simulink model "VirtualADAPT"  (Simulink 8.9)
settings: Solver=ode45  StartTime=0.0  StopTime=inf  RelTol=1e-3  EnableMultiTasking=on
PostLoadFcn:
  | FaultInjectionGUIHandle = FaultInjectionGUI;
InitFcn:
  | VirtualADAPTDataStructure
undefined symbols (not defined in the file or in its folder): ln
libraries: simulink x363
(part 14 of 26)

== system VirtualADAPT/VirtualADAPTv1/PowerStorage/Battery2Subsystem/CircuitBreakerEY236/Delay ==
  Input : Inport
  C : Subsystem  mask{C=0.8, IC=0, FP=Nominal, FT=0, FM=0}
      mask prompts: C "Value of C:"; IC "Initial Condition:"; FP "Fault Profile:"; FT "Fault Injection Time:"; FM "Fault Magnitude/Slope"
      mask TypeOptions FP: Nominal | Abrupt | Incipient
      mask Display: image(imread('C.png','BackgroundColor',[1 1 1]))
  Function : Subsystem
      mask Display: image(imread('fx.png','BackgroundColor',[1 1 1]))
  OneJunction : Subsystem
      mask Display: image(imread('1.png','BackgroundColor',[1 1 1]))
  R : Subsystem  mask{R=1, FP=Nominal, FT=0, FM=0}
      mask prompts: R "Value of R:"; FP "Fault Profile:"; FT "Fault Injection Time:"; FM "Fault Magnitude/Slope"
      mask TypeOptions FP: Nominal | Abrupt | Incipient
      mask Display: image(imread('R.png','BackgroundColor',[1 1 1]))
  Se : Subsystem  mask{FP=Nominal, FT=0, FM=0}
      mask prompts: FP "Fault Profile:"; FT "Fault Injection Time:"; FM "Fault Magnitude/Slope/Bias/StuckAtValue"
      mask TypeOptions FP: Nominal | Abrupt | Incipient | Bias | StuckAt
      mask Display: image(imread('MSe.png','BackgroundColor',[1 1 1]))
  ZeroJunction : Subsystem
      mask Display: image(imread('0.png','BackgroundColor',[1 1 1]))
  Output : Outport
  wires:
  OneJunction/1(y(1)) -> R/1(In)
  R/1(Out) -> OneJunction/1(u(1))
  Se/1(E) -> OneJunction/2(u(2))
  OneJunction/2(y(2)) -> Se/1(F)
  ZeroJunction/1(y(1)) -> C/1(F)
  C/1(E) -> ZeroJunction/1(u(1))
  OneJunction/3(y(3)) -> ZeroJunction/2(u(2))
  ZeroJunction/2(y(2)) -> OneJunction/3(u(3))
  Function/1(y(1)) -> Se/2(Se)
  Input/1 -> Function/1(u(1))
  ZeroJunction/3(O) -> Output/1

== system VirtualADAPT/VirtualADAPTv1/PowerStorage/Battery2Subsystem/CircuitBreakerEY236/ParallelConn ==
  u(1) : Inport
  u(2) : Inport  Port=2
  u(3) : Inport  Port=3
  u(4) : Inport  Port=4
  u(5) : Inport  Port=5
  Adder : Sum  Inputs=--  SaturateOnIntegerOverflow=on  InputSameDT=on  OutDataTypeStr=Inherit: Same as first input
  Gain : Gain  Gain=-1  SaturateOnIntegerOverflow=on  OutDataTypeStr=Inherit: Same as input  ParamDataTypeStr=Inherit: Same as input
  Mux : Mux  Inputs=5
  OffGuard : Fcn  Expr=u(5)
  OnGuard : Fcn  Expr=u(4)
  Product1 : Product  SaturateOnIntegerOverflow=on  RndMeth=Zero  InputSameDT=on  OutDataTypeStr=Inherit: Same as first input
  Product2 : Product  SaturateOnIntegerOverflow=on  RndMeth=Zero  InputSameDT=on  OutDataTypeStr=Inherit: Same as first input
  determineState : Subsystem
  y(1) : Outport
  y(2) : Outport  Port=2
  y(3) : Outport  Port=3
  O : Outport  Port=4
  State : Outport  Port=5
  wires:
  Adder/1 -> Gain/1
  u(4)/1 -> Mux/4
  u(5)/1 -> Mux/5
  Mux/1 -> OnGuard/1, OffGuard/1
  OnGuard/1 -> determineState/1(In1)
  OffGuard/1 -> determineState/2(In2)
  u(1)/1 -> Product1/1
  determineState/1(Out1) -> Product1/2, Product2/2, State/1
  Product1/1 -> O/1, y(2)/1, y(3)/1
  Gain/1 -> Product2/1
  Product2/1 -> y(1)/1
  u(2)/1 -> Adder/1
  u(3)/1 -> Adder/2

== system VirtualADAPT/VirtualADAPTv1/PowerStorage/Battery2Subsystem/CircuitBreakerEY236/R ==
  In : Inport
  Bond : Constant  Value=2
  Computing R : Subsystem
  Fault Profile : Constant  Value=FP
  JuncDB : Constant  Value=3
  Junction Type : Constant  Value=0
  Ramp : simulink/Sources/Ramp  slope=FM  start=FT  X0=R
  Resistor Value : Constant  Value=R
  Step : Step  Time=FT  Before=R  After=(1 + FM)*R  SampleTime=0
  Out : Outport
  R : Outport  Port=2
  wires:
  Computing R/1(Out1) -> Out/1
  In/1 -> Computing R/1(In1)
  Resistor Value/1 -> Computing R/2(RNominal)
  Fault Profile/1 -> Computing R/3(Fault Profile)
  Step/1 -> Computing R/4(Step)
  Ramp/1 -> Computing R/5(Ramp)
  JuncDB/1 -> Computing R/6(JuncDB)
  Bond/1 -> Computing R/7(Bond)
  Junction Type/1 -> Computing R/8(Junction Type)
  Computing R/2(Out2) -> R/1

== system VirtualADAPT/VirtualADAPTv1/PowerStorage/Battery2Subsystem/CircuitBreakerEY236/ZeroJunc ==
  u(1) : Inport
  u(2) : Inport  Port=2
  u(3) : Inport  Port=3
  Adder : Sum  Inputs=+-  SaturateOnIntegerOverflow=on  InputSameDT=on  OutDataTypeStr=Inherit: Same as first input
  Gain : Gain  SaturateOnIntegerOverflow=on  OutDataTypeStr=Inherit: Same as input  ParamDataTypeStr=Inherit: Same as input
  y(1) : Outport
  y(2) : Outport  Port=2
  y(3) : Outport  Port=3
  O : Outport  Port=4
  wires:
  Adder/1 -> Gain/1
  u(3)/1 -> y(1)/1, y(2)/1, O/1
  u(1)/1 -> Adder/1
  u(2)/1 -> Adder/2
  Gain/1 -> y(3)/1

== system VirtualADAPT/VirtualADAPTv1/PowerStorage/Battery2Subsystem/RelayEY241/ParallelConn ==
  u(1) : Inport
  u(2) : Inport  Port=2
  u(3) : Inport  Port=3
  Adder : Sum  Inputs=-  SaturateOnIntegerOverflow=on  InputSameDT=on  OutDataTypeStr=Inherit: Same as first input
  Gain : Gain  Gain=-1  SaturateOnIntegerOverflow=on  OutDataTypeStr=Inherit: Same as input  ParamDataTypeStr=Inherit: Same as input
  Mux : Mux  Inputs=3
  OffGuard : Fcn  Expr=(!u(3))
  OnGuard : Fcn  Expr=(u(3))
  Product1 : Product  SaturateOnIntegerOverflow=on  RndMeth=Zero  InputSameDT=on  OutDataTypeStr=Inherit: Same as first input
  Product2 : Product  SaturateOnIntegerOverflow=on  RndMeth=Zero  InputSameDT=on  OutDataTypeStr=Inherit: Same as first input
  determineState : Subsystem
  y(1) : Outport
  y(2) : Outport  Port=2
  O : Outport  Port=3
  State : Outport  Port=4
  wires:
  Adder/1 -> Gain/1
  u(3)/1 -> Mux/3
  Mux/1 -> OnGuard/1, OffGuard/1
  OnGuard/1 -> determineState/1(In1)
  OffGuard/1 -> determineState/2(In2)
  u(1)/1 -> Product1/1
  determineState/1(Out1) -> Product1/2, Product2/2, State/1
  Product1/1 -> O/1, y(2)/1
  Gain/1 -> Product2/1
  Product2/1 -> y(1)/1
  u(2)/1 -> Adder/1

== system VirtualADAPT/VirtualADAPTv1/PowerStorage/Battery2Subsystem/RelayEY241/Relay ==
  u(1) : Inport
  u(2) : Inport  Port=2
  u(3) : Inport  Port=3
  Adder : Sum  Inputs=+  SaturateOnIntegerOverflow=on  InputSameDT=on  OutDataTypeStr=Inherit: Same as first input
  Gain : Gain  SaturateOnIntegerOverflow=on  OutDataTypeStr=Inherit: Same as input  ParamDataTypeStr=Inherit: Same as input
  Mux : Mux  Inputs=3
  OffGuard : Fcn  Expr=(!u(3))
  OnGuard : Fcn  Expr=(u(3))
  Product1 : Product  SaturateOnIntegerOverflow=on  RndMeth=Zero  InputSameDT=on  OutDataTypeStr=Inherit: Same as first input
  Product2 : Product  SaturateOnIntegerOverflow=on  RndMeth=Zero  InputSameDT=on  OutDataTypeStr=Inherit: Same as first input
  determineState : Subsystem
  y(1) : Outport
  y(2) : Outport  Port=2
  O : Outport  Port=3
  State : Outport  Port=4
  wires:
  Adder/1 -> Gain/1
  u(3)/1 -> Mux/3
  Mux/1 -> OnGuard/1, OffGuard/1
  OnGuard/1 -> determineState/1(In1)
  OffGuard/1 -> determineState/2(In2)
  u(1)/1 -> Product1/1
  determineState/1(Out1) -> Product1/2, Product2/2, State/1
  Product1/1 -> O/1, y(2)/1
  Gain/1 -> Product2/1
  Product2/1 -> y(1)/1
  u(2)/1 -> Adder/1

== system VirtualADAPT/VirtualADAPTv1/PowerStorage/Battery2Subsystem/RelayEY241/Sensor ==
  TrueValue : Inport
  MSe : Subsystem  mask{FP=Nominal, FT=0, FM=0}
      mask prompts: FP "Fault Profile:"; FT "Fault Injection Time:"; FM "Fault Magnitude/Slope/Bias/StuckAtValue"
      mask TypeOptions FP: Nominal | Abrupt | Incipient | Bias | StuckAt
      mask Display: image(imread('MSe.png','BackgroundColor',[1 1 1]))
  R : Subsystem  mask{R=1, FP=Nominal, FT=0, FM=0}
      mask prompts: R "Value of R:"; FP "Fault Profile:"; FT "Fault Injection Time:"; FM "Fault Magnitude/Slope"
      mask TypeOptions FP: Nominal | Abrupt | Incipient
      mask Display: image(imread('R.png','BackgroundColor',[1 1 1]))
  TrueValueMod : Subsystem
      mask Display: image(imread('fx.png','BackgroundColor',[1 1 1]))
  Value : Subsystem
      mask Display: image(imread('0.png','BackgroundColor',[1 1 1]))
  SensedValue : Outport
  wires:
  Value/1(y(1)) -> R/1(In)
  R/1(Out) -> Value/1(u(1))
  MSe/1(E) -> Value/2(u(2))
  Value/2(y(2)) -> MSe/1(F)
  TrueValueMod/1(y(1)) -> MSe/2(Se)
  TrueValue/1 -> TrueValueMod/1(u(1))
  Value/3(O) -> SensedValue/1

== system VirtualADAPT/VirtualADAPTv1/PowerStorage/Battery2Subsystem/RelayEY244/ParallelConn ==
  u(1) : Inport
  u(2) : Inport  Port=2
  u(3) : Inport  Port=3
  Adder : Sum  Inputs=-  SaturateOnIntegerOverflow=on  InputSameDT=on  OutDataTypeStr=Inherit: Same as first input
  Gain : Gain  Gain=-1  SaturateOnIntegerOverflow=on  OutDataTypeStr=Inherit: Same as input  ParamDataTypeStr=Inherit: Same as input
  Mux : Mux  Inputs=3
  OffGuard : Fcn  Expr=(!u(3))
  OnGuard : Fcn  Expr=(u(3))
  Product1 : Product  SaturateOnIntegerOverflow=on  RndMeth=Zero  InputSameDT=on  OutDataTypeStr=Inherit: Same as first input
  Product2 : Product  SaturateOnIntegerOverflow=on  RndMeth=Zero  InputSameDT=on  OutDataTypeStr=Inherit: Same as first input
  determineState : Subsystem
  y(1) : Outport
  y(2) : Outport  Port=2
  O : Outport  Port=3
  State : Outport  Port=4
  wires:
  Adder/1 -> Gain/1
  u(3)/1 -> Mux/3
  Mux/1 -> OnGuard/1, OffGuard/1
  OnGuard/1 -> determineState/1(In1)
  OffGuard/1 -> determineState/2(In2)
  u(1)/1 -> Product1/1
  determineState/1(Out1) -> Product1/2, Product2/2, State/1
  Product1/1 -> O/1, y(2)/1
  Gain/1 -> Product2/1
  Product2/1 -> y(1)/1
  u(2)/1 -> Adder/1

== system VirtualADAPT/VirtualADAPTv1/PowerStorage/Battery2Subsystem/RelayEY244/Relay ==
  u(1) : Inport
  u(2) : Inport  Port=2
  u(3) : Inport  Port=3
  Adder : Sum  Inputs=+  SaturateOnIntegerOverflow=on  InputSameDT=on  OutDataTypeStr=Inherit: Same as first input
  Gain : Gain  SaturateOnIntegerOverflow=on  OutDataTypeStr=Inherit: Same as input  ParamDataTypeStr=Inherit: Same as input
  Mux : Mux  Inputs=3
  OffGuard : Fcn  Expr=(!u(3))
  OnGuard : Fcn  Expr=(u(3))
  Product1 : Product  SaturateOnIntegerOverflow=on  RndMeth=Zero  InputSameDT=on  OutDataTypeStr=Inherit: Same as first input
  Product2 : Product  SaturateOnIntegerOverflow=on  RndMeth=Zero  InputSameDT=on  OutDataTypeStr=Inherit: Same as first input
  determineState : Subsystem
  y(1) : Outport
  y(2) : Outport  Port=2
  O : Outport  Port=3
  State : Outport  Port=4
  wires:
  Adder/1 -> Gain/1
  u(3)/1 -> Mux/3
  Mux/1 -> OnGuard/1, OffGuard/1
  OnGuard/1 -> determineState/1(In1)
  OffGuard/1 -> determineState/2(In2)
  u(1)/1 -> Product1/1
  determineState/1(Out1) -> Product1/2, Product2/2, State/1
  Product1/1 -> O/1, y(2)/1
  Gain/1 -> Product2/1
  Product2/1 -> y(1)/1
  u(2)/1 -> Adder/1

== system VirtualADAPT/VirtualADAPTv1/PowerStorage/Battery2Subsystem/RelayEY244/Sensor ==
  TrueValue : Inport
  MSe : Subsystem  mask{FP=Nominal, FT=0, FM=0}
      mask prompts: FP "Fault Profile:"; FT "Fault Injection Time:"; FM "Fault Magnitude/Slope/Bias/StuckAtValue"
      mask TypeOptions FP: Nominal | Abrupt | Incipient | Bias | StuckAt
      mask Display: image(imread('MSe.png','BackgroundColor',[1 1 1]))
  R : Subsystem  mask{R=1, FP=Nominal, FT=0, FM=0}
      mask prompts: R "Value of R:"; FP "Fault Profile:"; FT "Fault Injection Time:"; FM "Fault Magnitude/Slope"
      mask TypeOptions FP: Nominal | Abrupt | Incipient
      mask Display: image(imread('R.png','BackgroundColor',[1 1 1]))
  TrueValueMod : Subsystem
      mask Display: image(imread('fx.png','BackgroundColor',[1 1 1]))
  Value : Subsystem
      mask Display: image(imread('0.png','BackgroundColor',[1 1 1]))
  SensedValue : Outport
  wires:
  Value/1(y(1)) -> R/1(In)
  R/1(Out) -> Value/1(u(1))
  MSe/1(E) -> Value/2(u(2))
  Value/2(y(2)) -> MSe/1(F)
  TrueValueMod/1(y(1)) -> MSe/2(Se)
  TrueValue/1 -> TrueValueMod/1(u(1))
  Value/3(O) -> SensedValue/1

== system VirtualADAPT/VirtualADAPTv1/PowerStorage/Battery2Subsystem/SensorE235/MSe ==
  F : Inport
  Se : Inport  Port=2
  Bias : Step  Time=FT  After=FM  SampleTime=0
  Fault Inject : Subsystem
  Fault Profile : Constant  Value=FP
  Mult2 : Product  SaturateOnIntegerOverflow=on  RndMeth=Zero  InputSameDT=on  OutDataTypeStr=Inherit: Same as first input
  Multiply : Product  SaturateOnIntegerOverflow=on  RndMeth=Zero  InputSameDT=on  OutDataTypeStr=Inherit: Same as first input
  Ramp : simulink/Sources/Ramp  slope=FM  start=FT  X0=0
  Step : Step  Time=FT  Before=1  After=(1 + FM)  SampleTime=0
  StuckAt : Step  Time=FT  Before=1  After=0  SampleTime=0
  StuckAt2 : Step  Time=FT  After=FM  SampleTime=0
  Sum : Sum  SaturateOnIntegerOverflow=on  InputSameDT=on  OutDataTypeStr=Inherit: Same as first input
  Sum2 : Sum  SaturateOnIntegerOverflow=on  InputSameDT=on  OutDataTypeStr=Inherit: Same as first input
  Sum3 : Sum  SaturateOnIntegerOverflow=on  InputSameDT=on  OutDataTypeStr=Inherit: Same as first input
  E : Outport
  wires:
  Fault Inject/1(Out1) -> E/1
  Se/1 -> Fault Inject/1(Nominal), Multiply/1, Sum/1, Sum2/1, Mult2/1
  Fault Profile/1 -> Fault Inject/2(Fault Profile)
  Step/1 -> Multiply/2
  Ramp/1 -> Sum/2
  Multiply/1 -> Fault Inject/3(Step)
  Sum/1 -> Fault Inject/4(Ramp)
  Bias/1 -> Sum2/2
  Sum2/1 -> Fault Inject/5(Bias)
  StuckAt/1 -> Mult2/2
  Mult2/1 -> Sum3/1
  StuckAt2/1 -> Sum3/2
  Sum3/1 -> Fault Inject/6(stuckAt)

== system VirtualADAPT/VirtualADAPTv1/PowerStorage/Battery2Subsystem/SensorE235/R ==
  In : Inport
  Bond : Constant  Value=2
  Computing R : Subsystem
  Fault Profile : Constant  Value=FP
  JuncDB : Constant
  Junction Type : Constant  Value=0
  Ramp : simulink/Sources/Ramp  slope=FM  start=FT  X0=R
  Resistor Value : Constant  Value=R
  Step : Step  Time=FT  Before=R  After=(1 + FM)*R  SampleTime=0
  Out : Outport
  R : Outport  Port=2
  wires:
  Computing R/1(Out1) -> Out/1
  In/1 -> Computing R/1(In1)
  Resistor Value/1 -> Computing R/2(RNominal)
  Fault Profile/1 -> Computing R/3(Fault Profile)
  Step/1 -> Computing R/4(Step)
  Ramp/1 -> Computing R/5(Ramp)
  JuncDB/1 -> Computing R/6(JuncDB)
  Bond/1 -> Computing R/7(Bond)
  Junction Type/1 -> Computing R/8(Junction Type)
  Computing R/2(Out2) -> R/1

== system VirtualADAPT/VirtualADAPTv1/PowerStorage/Battery2Subsystem/SensorE235/TrueValueMod ==
  u(1) : Inport
  Function : Fcn  Expr=u(1)
  Mux : Mux  Inputs=1
  y(1) : Outport
  wires:
  u(1)/1 -> Mux/1
  Mux/1 -> Function/1
  Function/1 -> y(1)/1

== system VirtualADAPT/VirtualADAPTv1/PowerStorage/Battery2Subsystem/SensorE235/Value ==
  u(1) : Inport
  u(2) : Inport  Port=2
  Adder : Sum  Inputs=-  SaturateOnIntegerOverflow=on  InputSameDT=on  OutDataTypeStr=Inherit: Same as first input
  Gain : Gain  Gain=-1  SaturateOnIntegerOverflow=on  OutDataTypeStr=Inherit: Same as input  ParamDataTypeStr=Inherit: Same as input
  y(1) : Outport
  y(2) : Outport  Port=2
  O : Outport  Port=3
  wires:
  Adder/1 -> Gain/1
  Gain/1 -> y(1)/1
  u(1)/1 -> y(2)/1, O/1
  u(2)/1 -> Adder/1

== system VirtualADAPT/VirtualADAPTv1/PowerStorage/Battery2Subsystem/SensorE240/MSe ==
  F : Inport
  Se : Inport  Port=2
  Bias : Step  Time=FT  After=FM  SampleTime=0
  Fault Inject : Subsystem
  Fault Profile : Constant  Value=FP
  Mult2 : Product  SaturateOnIntegerOverflow=on  RndMeth=Zero  InputSameDT=on  OutDataTypeStr=Inherit: Same as first input
  Multiply : Product  SaturateOnIntegerOverflow=on  RndMeth=Zero  InputSameDT=on  OutDataTypeStr=Inherit: Same as first input
  Ramp : simulink/Sources/Ramp  slope=FM  start=FT  X0=0
  Step : Step  Time=FT  Before=1  After=(1 + FM)  SampleTime=0
  StuckAt : Step  Time=FT  Before=1  After=0  SampleTime=0
  StuckAt2 : Step  Time=FT  After=FM  SampleTime=0
  Sum : Sum  SaturateOnIntegerOverflow=on  InputSameDT=on  OutDataTypeStr=Inherit: Same as first input
  Sum2 : Sum  SaturateOnIntegerOverflow=on  InputSameDT=on  OutDataTypeStr=Inherit: Same as first input
  Sum3 : Sum  SaturateOnIntegerOverflow=on  InputSameDT=on  OutDataTypeStr=Inherit: Same as first input
  E : Outport
  wires:
  Fault Inject/1(Out1) -> E/1
  Se/1 -> Fault Inject/1(Nominal), Multiply/1, Sum/1, Sum2/1, Mult2/1
  Fault Profile/1 -> Fault Inject/2(Fault Profile)
  Step/1 -> Multiply/2
  Ramp/1 -> Sum/2
  Multiply/1 -> Fault Inject/3(Step)
  Sum/1 -> Fault Inject/4(Ramp)
  Bias/1 -> Sum2/2
  Sum2/1 -> Fault Inject/5(Bias)
  StuckAt/1 -> Mult2/2
  Mult2/1 -> Sum3/1
  StuckAt2/1 -> Sum3/2
  Sum3/1 -> Fault Inject/6(stuckAt)

== system VirtualADAPT/VirtualADAPTv1/PowerStorage/Battery2Subsystem/SensorE240/R ==
  In : Inport
  Bond : Constant
  Computing R : Subsystem
  Fault Profile : Constant  Value=FP
  JuncDB : Constant  Value=2
  Junction Type : Constant  Value=0
  Ramp : simulink/Sources/Ramp  slope=FM  start=FT  X0=R
  Resistor Value : Constant  Value=R
  Step : Step  Time=FT  Before=R  After=(1 + FM)*R  SampleTime=0
  Out : Outport
  R : Outport  Port=2
  wires:
  Computing R/1(Out1) -> Out/1
  In/1 -> Computing R/1(In1)
  Resistor Value/1 -> Computing R/2(RNominal)
  Fault Profile/1 -> Computing R/3(Fault Profile)
  Step/1 -> Computing R/4(Step)
  Ramp/1 -> Computing R/5(Ramp)
  JuncDB/1 -> Computing R/6(JuncDB)
  Bond/1 -> Computing R/7(Bond)
  Junction Type/1 -> Computing R/8(Junction Type)
  Computing R/2(Out2) -> R/1

== system VirtualADAPT/VirtualADAPTv1/PowerStorage/Battery2Subsystem/SensorE240/TrueValueMod ==
  u(1) : Inport
  Function : Fcn  Expr=u(1)
  Mux : Mux  Inputs=1
  y(1) : Outport
  wires:
  u(1)/1 -> Mux/1
  Mux/1 -> Function/1
  Function/1 -> y(1)/1

== system VirtualADAPT/VirtualADAPTv1/PowerStorage/Battery2Subsystem/SensorE240/Value ==
  u(1) : Inport
  u(2) : Inport  Port=2
  Adder : Sum  Inputs=-  SaturateOnIntegerOverflow=on  InputSameDT=on  OutDataTypeStr=Inherit: Same as first input
  Gain : Gain  Gain=-1  SaturateOnIntegerOverflow=on  OutDataTypeStr=Inherit: Same as input  ParamDataTypeStr=Inherit: Same as input
  y(1) : Outport
  y(2) : Outport  Port=2
  O : Outport  Port=3
  wires:
  Adder/1 -> Gain/1
  u(2)/1 -> y(1)/1, O/1
  u(1)/1 -> Adder/1
  Gain/1 -> y(2)/1

== system VirtualADAPT/VirtualADAPTv1/PowerStorage/Battery2Subsystem/SensorE242/MSe ==
  F : Inport
  Se : Inport  Port=2
  Bias : Step  Time=FT  After=FM  SampleTime=0
  Fault Inject : Subsystem
  Fault Profile : Constant  Value=FP
  Mult2 : Product  SaturateOnIntegerOverflow=on  RndMeth=Zero  InputSameDT=on  OutDataTypeStr=Inherit: Same as first input
  Multiply : Product  SaturateOnIntegerOverflow=on  RndMeth=Zero  InputSameDT=on  OutDataTypeStr=Inherit: Same as first input
  Ramp : simulink/Sources/Ramp  slope=FM  start=FT  X0=0
  Step : Step  Time=FT  Before=1  After=(1 + FM)  SampleTime=0
  StuckAt : Step  Time=FT  Before=1  After=0  SampleTime=0
  StuckAt2 : Step  Time=FT  After=FM  SampleTime=0
  Sum : Sum  SaturateOnIntegerOverflow=on  InputSameDT=on  OutDataTypeStr=Inherit: Same as first input
  Sum2 : Sum  SaturateOnIntegerOverflow=on  InputSameDT=on  OutDataTypeStr=Inherit: Same as first input
  Sum3 : Sum  SaturateOnIntegerOverflow=on  InputSameDT=on  OutDataTypeStr=Inherit: Same as first input
  E : Outport
  wires:
  Fault Inject/1(Out1) -> E/1
  Se/1 -> Fault Inject/1(Nominal), Multiply/1, Sum/1, Sum2/1, Mult2/1
  Fault Profile/1 -> Fault Inject/2(Fault Profile)
  Step/1 -> Multiply/2
  Ramp/1 -> Sum/2
  Multiply/1 -> Fault Inject/3(Step)
  Sum/1 -> Fault Inject/4(Ramp)
  Bias/1 -> Sum2/2
  Sum2/1 -> Fault Inject/5(Bias)
  StuckAt/1 -> Mult2/2
  Mult2/1 -> Sum3/1
  StuckAt2/1 -> Sum3/2
  Sum3/1 -> Fault Inject/6(stuckAt)

== system VirtualADAPT/VirtualADAPTv1/PowerStorage/Battery2Subsystem/SensorE242/R ==
  In : Inport
  Bond : Constant
  Computing R : Subsystem
  Fault Profile : Constant  Value=FP
  JuncDB : Constant  Value=2
  Junction Type : Constant  Value=0
  Ramp : simulink/Sources/Ramp  slope=FM  start=FT  X0=R
  Resistor Value : Constant  Value=R
  Step : Step  Time=FT  Before=R  After=(1 + FM)*R  SampleTime=0
  Out : Outport
  R : Outport  Port=2
  wires:
  Computing R/1(Out1) -> Out/1
  In/1 -> Computing R/1(In1)
  Resistor Value/1 -> Computing R/2(RNominal)
  Fault Profile/1 -> Computing R/3(Fault Profile)
  Step/1 -> Computing R/4(Step)
  Ramp/1 -> Computing R/5(Ramp)
  JuncDB/1 -> Computing R/6(JuncDB)
  Bond/1 -> Computing R/7(Bond)
  Junction Type/1 -> Computing R/8(Junction Type)
  Computing R/2(Out2) -> R/1

== system VirtualADAPT/VirtualADAPTv1/PowerStorage/Battery2Subsystem/SensorE242/TrueValueMod ==
  u(1) : Inport
  Function : Fcn  Expr=u(1)
  Mux : Mux  Inputs=1
  y(1) : Outport
  wires:
  u(1)/1 -> Mux/1
  Mux/1 -> Function/1
  Function/1 -> y(1)/1

== system VirtualADAPT/VirtualADAPTv1/PowerStorage/Battery2Subsystem/SensorE242/Value ==
  u(1) : Inport
  u(2) : Inport  Port=2
  Adder : Sum  Inputs=-  SaturateOnIntegerOverflow=on  InputSameDT=on  OutDataTypeStr=Inherit: Same as first input
  Gain : Gain  Gain=-1  SaturateOnIntegerOverflow=on  OutDataTypeStr=Inherit: Same as input  ParamDataTypeStr=Inherit: Same as input
  y(1) : Outport
  y(2) : Outport  Port=2
  O : Outport  Port=3
  wires:
  Adder/1 -> Gain/1
  u(2)/1 -> y(1)/1, O/1
  u(1)/1 -> Adder/1
  Gain/1 -> y(2)/1

== system VirtualADAPT/VirtualADAPTv1/PowerStorage/Battery2Subsystem/SensorISH236/Function ==
  u(1) : Inport
  Function : Fcn  Expr=u(1)
  Mux : Mux  Inputs=1
  y(1) : Outport
  wires:
  u(1)/1 -> Mux/1
  Mux/1 -> Function/1
  Function/1 -> y(1)/1

== system VirtualADAPT/VirtualADAPTv1/PowerStorage/Battery2Subsystem/SensorISH236/MSe ==
  F : Inport
  Se : Inport  Port=2
  Bias : Step  Time=FT  After=FM  SampleTime=0
  Fault Inject : Subsystem
  Fault Profile : Constant  Value=FP
  Mult2 : Product  SaturateOnIntegerOverflow=on  RndMeth=Zero  InputSameDT=on  OutDataTypeStr=Inherit: Same as first input
  Multiply : Product  SaturateOnIntegerOverflow=on  RndMeth=Zero  InputSameDT=on  OutDataTypeStr=Inherit: Same as first input
  Ramp : simulink/Sources/Ramp  slope=FM  start=FT  X0=0
  Step : Step  Time=FT  Before=1  After=(1 + FM)  SampleTime=0
  StuckAt : Step  Time=FT  Before=1  After=0  SampleTime=0
  StuckAt2 : Step  Time=FT  After=FM  SampleTime=0
  Sum : Sum  SaturateOnIntegerOverflow=on  InputSameDT=on  OutDataTypeStr=Inherit: Same as first input
  Sum2 : Sum  SaturateOnIntegerOverflow=on  InputSameDT=on  OutDataTypeStr=Inherit: Same as first input
  Sum3 : Sum  SaturateOnIntegerOverflow=on  InputSameDT=on  OutDataTypeStr=Inherit: Same as first input
  E : Outport
  wires:
  Fault Inject/1(Out1) -> E/1
  Se/1 -> Fault Inject/1(Nominal), Multiply/1, Sum/1, Sum2/1, Mult2/1
  Fault Profile/1 -> Fault Inject/2(Fault Profile)
  Step/1 -> Multiply/2
  Ramp/1 -> Sum/2
  Multiply/1 -> Fault Inject/3(Step)
  Sum/1 -> Fault Inject/4(Ramp)
  Bias/1 -> Sum2/2
  Sum2/1 -> Fault Inject/5(Bias)
  StuckAt/1 -> Mult2/2
  Mult2/1 -> Sum3/1
  StuckAt2/1 -> Sum3/2
  Sum3/1 -> Fault Inject/6(stuckAt)

== system VirtualADAPT/VirtualADAPTv1/PowerStorage/Battery2Subsystem/SensorISH236/R ==
  In : Inport
  Bond : Constant  Value=2
  Computing R : Subsystem
  Fault Profile : Constant  Value=FP
  JuncDB : Constant
  Junction Type : Constant  Value=0
  Ramp : simulink/Sources/Ramp  slope=FM  start=FT  X0=R
  Resistor Value : Constant  Value=R
  Step : Step  Time=FT  Before=R  After=(1 + FM)*R  SampleTime=0
  Out : Outport
  R : Outport  Port=2
  wires:
  Computing R/1(Out1) -> Out/1
  In/1 -> Computing R/1(In1)
  Resistor Value/1 -> Computing R/2(RNominal)
  Fault Profile/1 -> Computing R/3(Fault Profile)
  Step/1 -> Computing R/4(Step)
  Ramp/1 -> Computing R/5(Ramp)
  JuncDB/1 -> Computing R/6(JuncDB)
  Bond/1 -> Computing R/7(Bond)
  Junction Type/1 -> Computing R/8(Junction Type)
  Computing R/2(Out2) -> R/1

== system VirtualADAPT/VirtualADAPTv1/PowerStorage/Battery2Subsystem/SensorISH236/Value ==
  u(1) : Inport
  u(2) : Inport  Port=2
  Adder : Sum  Inputs=-  SaturateOnIntegerOverflow=on  InputSameDT=on  OutDataTypeStr=Inherit: Same as first input
  Gain : Gain  Gain=-1  SaturateOnIntegerOverflow=on  OutDataTypeStr=Inherit: Same as input  ParamDataTypeStr=Inherit: Same as input
  y(1) : Outport
  y(2) : Outport  Port=2
  O : Outport  Port=3
  wires:
  Adder/1 -> Gain/1
  Gain/1 -> y(1)/1
  u(1)/1 -> y(2)/1, O/1
  u(2)/1 -> Adder/1

== system VirtualADAPT/VirtualADAPTv1/PowerStorage/Battery2Subsystem/SensorIT240/MSe ==
  F : Inport
  Se : Inport  Port=2
  Bias : Step  Time=FT  After=FM  SampleTime=0
  Fault Inject : Subsystem
  Fault Profile : Constant  Value=FP
  Mult2 : Product  SaturateOnIntegerOverflow=on  RndMeth=Zero  InputSameDT=on  OutDataTypeStr=Inherit: Same as first input
  Multiply : Product  SaturateOnIntegerOverflow=on  RndMeth=Zero  InputSameDT=on  OutDataTypeStr=Inherit: Same as first input
  Ramp : simulink/Sources/Ramp  slope=FM  start=FT  X0=0
  Step : Step  Time=FT  Before=1  After=(1 + FM)  SampleTime=0
  StuckAt : Step  Time=FT  Before=1  After=0  SampleTime=0
  StuckAt2 : Step  Time=FT  After=FM  SampleTime=0
  Sum : Sum  SaturateOnIntegerOverflow=on  InputSameDT=on  OutDataTypeStr=Inherit: Same as first input
  Sum2 : Sum  SaturateOnIntegerOverflow=on  InputSameDT=on  OutDataTypeStr=Inherit: Same as first input
  Sum3 : Sum  SaturateOnIntegerOverflow=on  InputSameDT=on  OutDataTypeStr=Inherit: Same as first input
  E : Outport
  wires:
  Fault Inject/1(Out1) -> E/1
  Se/1 -> Fault Inject/1(Nominal), Multiply/1, Sum/1, Sum2/1, Mult2/1
  Fault Profile/1 -> Fault Inject/2(Fault Profile)
  Step/1 -> Multiply/2
  Ramp/1 -> Sum/2
  Multiply/1 -> Fault Inject/3(Step)
  Sum/1 -> Fault Inject/4(Ramp)
  Bias/1 -> Sum2/2
  Sum2/1 -> Fault Inject/5(Bias)
  StuckAt/1 -> Mult2/2
  Mult2/1 -> Sum3/1
  StuckAt2/1 -> Sum3/2
  Sum3/1 -> Fault Inject/6(stuckAt)

== system VirtualADAPT/VirtualADAPTv1/PowerStorage/Battery2Subsystem/SensorIT240/R ==
  In : Inport
  Bond : Constant
  Computing R : Subsystem
  Fault Profile : Constant  Value=FP
  JuncDB : Constant  Value=2
  Junction Type : Constant  Value=0
  Ramp : simulink/Sources/Ramp  slope=FM  start=FT  X0=R
  Resistor Value : Constant  Value=R
  Step : Step  Time=FT  Before=R  After=(1 + FM)*R  SampleTime=0
  Out : Outport
  R : Outport  Port=2
  wires:
  Computing R/1(Out1) -> Out/1
  In/1 -> Computing R/1(In1)
  Resistor Value/1 -> Computing R/2(RNominal)
  Fault Profile/1 -> Computing R/3(Fault Profile)
  Step/1 -> Computing R/4(Step)
  Ramp/1 -> Computing R/5(Ramp)
  JuncDB/1 -> Computing R/6(JuncDB)
  Bond/1 -> Computing R/7(Bond)
  Junction Type/1 -> Computing R/8(Junction Type)
  Computing R/2(Out2) -> R/1

== system VirtualADAPT/VirtualADAPTv1/PowerStorage/Battery2Subsystem/SensorIT240/TrueValueMod ==
  u(1) : Inport
  Function : Fcn  Expr=u(1)
  Mux : Mux  Inputs=1
  y(1) : Outport
  wires:
  u(1)/1 -> Mux/1
  Mux/1 -> Function/1
  Function/1 -> y(1)/1

== system VirtualADAPT/VirtualADAPTv1/PowerStorage/Battery2Subsystem/SensorIT240/Value ==
  u(1) : Inport
  u(2) : Inport  Port=2
  Adder : Sum  Inputs=-  SaturateOnIntegerOverflow=on  InputSameDT=on  OutDataTypeStr=Inherit: Same as first input
  Gain : Gain  Gain=-1  SaturateOnIntegerOverflow=on  OutDataTypeStr=Inherit: Same as input  ParamDataTypeStr=Inherit: Same as input
  y(1) : Outport
  y(2) : Outport  Port=2
  O : Outport  Port=3
  wires:
  Adder/1 -> Gain/1
  u(2)/1 -> y(1)/1, O/1
  u(1)/1 -> Adder/1
  Gain/1 -> y(2)/1

== system VirtualADAPT/VirtualADAPTv1/PowerStorage/Battery2Subsystem/SensorTE228/MSe ==
  F : Inport
  Se : Inport  Port=2
  Bias : Step  Time=FT  After=FM  SampleTime=0
  Fault Inject : Subsystem
  Fault Profile : Constant  Value=FP
  Mult2 : Product  SaturateOnIntegerOverflow=on  RndMeth=Zero  InputSameDT=on  OutDataTypeStr=Inherit: Same as first input
  Multiply : Product  SaturateOnIntegerOverflow=on  RndMeth=Zero  InputSameDT=on  OutDataTypeStr=Inherit: Same as first input
  Ramp : simulink/Sources/Ramp  slope=FM  start=FT  X0=0
  Step : Step  Time=FT  Before=1  After=(1 + FM)  SampleTime=0
  StuckAt : Step  Time=FT  Before=1  After=0  SampleTime=0
  StuckAt2 : Step  Time=FT  After=FM  SampleTime=0
  Sum : Sum  SaturateOnIntegerOverflow=on  InputSameDT=on  OutDataTypeStr=Inherit: Same as first input
  Sum2 : Sum  SaturateOnIntegerOverflow=on  InputSameDT=on  OutDataTypeStr=Inherit: Same as first input
  Sum3 : Sum  SaturateOnIntegerOverflow=on  InputSameDT=on  OutDataTypeStr=Inherit: Same as first input
  E : Outport
  wires:
  Fault Inject/1(Out1) -> E/1
  Se/1 -> Fault Inject/1(Nominal), Multiply/1, Sum/1, Sum2/1, Mult2/1
  Fault Profile/1 -> Fault Inject/2(Fault Profile)
  Step/1 -> Multiply/2
  Ramp/1 -> Sum/2
  Multiply/1 -> Fault Inject/3(Step)
  Sum/1 -> Fault Inject/4(Ramp)
  Bias/1 -> Sum2/2
  Sum2/1 -> Fault Inject/5(Bias)
  StuckAt/1 -> Mult2/2
  Mult2/1 -> Sum3/1
  StuckAt2/1 -> Sum3/2
  Sum3/1 -> Fault Inject/6(stuckAt)

== system VirtualADAPT/VirtualADAPTv1/PowerStorage/Battery2Subsystem/SensorTE228/R ==
  In : Inport
  Bond : Constant
  Computing R : Subsystem
  Fault Profile : Constant  Value=FP
  JuncDB : Constant  Value=2
  Junction Type : Constant  Value=0
  Ramp : simulink/Sources/Ramp  slope=FM  start=FT  X0=R
  Resistor Value : Constant  Value=R
  Step : Step  Time=FT  Before=R  After=(1 + FM)*R  SampleTime=0
  Out : Outport
  R : Outport  Port=2
  wires:
  Computing R/1(Out1) -> Out/1
  In/1 -> Computing R/1(In1)
  Resistor Value/1 -> Computing R/2(RNominal)
  Fault Profile/1 -> Computing R/3(Fault Profile)
  Step/1 -> Computing R/4(Step)
  Ramp/1 -> Computing R/5(Ramp)
  JuncDB/1 -> Computing R/6(JuncDB)
  Bond/1 -> Computing R/7(Bond)
  Junction Type/1 -> Computing R/8(Junction Type)
  Computing R/2(Out2) -> R/1

== system VirtualADAPT/VirtualADAPTv1/PowerStorage/Battery2Subsystem/SensorTE228/TempBias ==
  Constant : Constant  Value=Constant
  Fault Inject : Subsystem
  Fault Profile : Constant  Value=FP
  Ramp : simulink/Sources/Ramp  slope=FM  start=FT  X0=Constant
  Step : Step  Time=FT  Before=Constant  After=(1 + FM)*Constant  SampleTime=0
  C : Outport
  wires:
  Fault Inject/1(Out1) -> C/1
  Constant/1 -> Fault Inject/1(Nominal)
  Fault Profile/1 -> Fault Inject/2(Fault Profile)
  Step/1 -> Fault Inject/3(Step)
  Ramp/1 -> Fault Inject/4(Ramp)

== system VirtualADAPT/VirtualADAPTv1/PowerStorage/Battery2Subsystem/SensorTE228/TrueValueMod ==
  u(1) : Inport
  u(2) : Inport  Port=2
  Function : Fcn  Expr=(u(1)+u(2))*9/5+32
  Mux : Mux  Inputs=2
  y(1) : Outport
  wires:
  u(1)/1 -> Mux/1
  u(2)/1 -> Mux/2
  Mux/1 -> Function/1
  Function/1 -> y(1)/1

== system VirtualADAPT/VirtualADAPTv1/PowerStorage/Battery2Subsystem/SensorTE228/Value ==
  u(1) : Inport
  u(2) : Inport  Port=2
  Adder : Sum  Inputs=-  SaturateOnIntegerOverflow=on  InputSameDT=on  OutDataTypeStr=Inherit: Same as first input
  Gain : Gain  Gain=-1  SaturateOnIntegerOverflow=on  OutDataTypeStr=Inherit: Same as input  ParamDataTypeStr=Inherit: Same as input
  y(1) : Outport
  y(2) : Outport  Port=2
  O : Outport  Port=3
  wires:
  Adder/1 -> Gain/1
  u(2)/1 -> y(1)/1, O/1
  u(1)/1 -> Adder/1
  Gain/1 -> y(2)/1

== system VirtualADAPT/VirtualADAPTv1/PowerStorage/Battery2Subsystem/SensorTE229/MSe ==
  F : Inport
  Se : Inport  Port=2
  Bias : Step  Time=FT  After=FM  SampleTime=0
  Fault Inject : Subsystem
  Fault Profile : Constant  Value=FP
  Mult2 : Product  SaturateOnIntegerOverflow=on  RndMeth=Zero  InputSameDT=on  OutDataTypeStr=Inherit: Same as first input
  Multiply : Product  SaturateOnIntegerOverflow=on  RndMeth=Zero  InputSameDT=on  OutDataTypeStr=Inherit: Same as first input
  Ramp : simulink/Sources/Ramp  slope=FM  start=FT  X0=0
  Step : Step  Time=FT  Before=1  After=(1 + FM)  SampleTime=0
  StuckAt : Step  Time=FT  Before=1  After=0  SampleTime=0
  StuckAt2 : Step  Time=FT  After=FM  SampleTime=0
  Sum : Sum  SaturateOnIntegerOverflow=on  InputSameDT=on  OutDataTypeStr=Inherit: Same as first input
  Sum2 : Sum  SaturateOnIntegerOverflow=on  InputSameDT=on  OutDataTypeStr=Inherit: Same as first input
  Sum3 : Sum  SaturateOnIntegerOverflow=on  InputSameDT=on  OutDataTypeStr=Inherit: Same as first input
  E : Outport
  wires:
  Fault Inject/1(Out1) -> E/1
  Se/1 -> Fault Inject/1(Nominal), Multiply/1, Sum/1, Sum2/1, Mult2/1
  Fault Profile/1 -> Fault Inject/2(Fault Profile)
  Step/1 -> Multiply/2
  Ramp/1 -> Sum/2
  Multiply/1 -> Fault Inject/3(Step)
  Sum/1 -> Fault Inject/4(Ramp)
  Bias/1 -> Sum2/2
  Sum2/1 -> Fault Inject/5(Bias)
  StuckAt/1 -> Mult2/2
  Mult2/1 -> Sum3/1
  StuckAt2/1 -> Sum3/2
  Sum3/1 -> Fault Inject/6(stuckAt)

== system VirtualADAPT/VirtualADAPTv1/PowerStorage/Battery2Subsystem/SensorTE229/R ==
  In : Inport
  Bond : Constant
  Computing R : Subsystem
  Fault Profile : Constant  Value=FP
  JuncDB : Constant  Value=2
  Junction Type : Constant  Value=0
  Ramp : simulink/Sources/Ramp  slope=FM  start=FT  X0=R
  Resistor Value : Constant  Value=R
  Step : Step  Time=FT  Before=R  After=(1 + FM)*R  SampleTime=0
  Out : Outport
  R : Outport  Port=2
  wires:
  Computing R/1(Out1) -> Out/1
  In/1 -> Computing R/1(In1)
  Resistor Value/1 -> Computing R/2(RNominal)
  Fault Profile/1 -> Computing R/3(Fault Profile)
  Step/1 -> Computing R/4(Step)
  Ramp/1 -> Computing R/5(Ramp)
  JuncDB/1 -> Computing R/6(JuncDB)
  Bond/1 -> Computing R/7(Bond)
  Junction Type/1 -> Computing R/8(Junction Type)
  Computing R/2(Out2) -> R/1

== system VirtualADAPT/VirtualADAPTv1/PowerStorage/Battery2Subsystem/SensorTE229/TempBias ==
  Constant : Constant  Value=Constant
  Fault Inject : Subsystem
  Fault Profile : Constant  Value=FP
  Ramp : simulink/Sources/Ramp  slope=FM  start=FT  X0=Constant
  Step : Step  Time=FT  Before=Constant  After=(1 + FM)*Constant  SampleTime=0
  C : Outport
  wires:
  Fault Inject/1(Out1) -> C/1
  Constant/1 -> Fault Inject/1(Nominal)
  Fault Profile/1 -> Fault Inject/2(Fault Profile)
  Step/1 -> Fault Inject/3(Step)
  Ramp/1 -> Fault Inject/4(Ramp)

== system VirtualADAPT/VirtualADAPTv1/PowerStorage/Battery2Subsystem/SensorTE229/TrueValueMod ==
  u(1) : Inport
  u(2) : Inport  Port=2
  Function : Fcn  Expr=(u(1)+u(2))*9/5+32
  Mux : Mux  Inputs=2
  y(1) : Outport
  wires:
  u(1)/1 -> Mux/1
  u(2)/1 -> Mux/2
  Mux/1 -> Function/1
  Function/1 -> y(1)/1

== system VirtualADAPT/VirtualADAPTv1/PowerStorage/Battery2Subsystem/SensorTE229/Value ==
  u(1) : Inport
  u(2) : Inport  Port=2
  Adder : Sum  Inputs=-  SaturateOnIntegerOverflow=on  InputSameDT=on  OutDataTypeStr=Inherit: Same as first input
  Gain : Gain  Gain=-1  SaturateOnIntegerOverflow=on  OutDataTypeStr=Inherit: Same as input  ParamDataTypeStr=Inherit: Same as input
  y(1) : Outport
  y(2) : Outport  Port=2
  O : Outport  Port=3
  wires:
  Adder/1 -> Gain/1
  u(2)/1 -> y(1)/1, O/1
  u(1)/1 -> Adder/1
  Gain/1 -> y(2)/1

== system VirtualADAPT/VirtualADAPTv1/PowerStorage/RelayEY160/ParallelConn/determineState ==
  In1 : Inport
  In2 : Inport  Port=2
  Fcn : Fcn  Expr=(!u(1)&&u(2)) || (u(1)&&(!u(3)))
  Memory : Memory
  Mux : Mux  Inputs=3
  Out1 : Outport
  wires:
  Memory/1 -> Mux/1
  In1/1 -> Mux/2
  In2/1 -> Mux/3
  Mux/1 -> Fcn/1
  Fcn/1 -> Memory/1, Out1/1

== system VirtualADAPT/VirtualADAPTv1/PowerStorage/RelayEY160/Relay/determineState ==
  In1 : Inport
  In2 : Inport  Port=2
  Fcn : Fcn  Expr=(!u(1)&&u(2)) || (u(1)&&(!u(3)))
  Memory : Memory
  Mux : Mux  Inputs=3
  Out1 : Outport
  wires:
  Memory/1 -> Mux/1
  In1/1 -> Mux/2
  In2/1 -> Mux/3
  Mux/1 -> Fcn/1
  Fcn/1 -> Memory/1, Out1/1

== system VirtualADAPT/VirtualADAPTv1/PowerStorage/RelayEY160/Sensor/MSe ==
  F : Inport
  Se : Inport  Port=2
  Bias : Step  Time=FT  After=FM  SampleTime=0
  Fault Inject : Subsystem
  Fault Profile : Constant  Value=FP
  Mult2 : Product  SaturateOnIntegerOverflow=on  RndMeth=Zero  InputSameDT=on  OutDataTypeStr=Inherit: Same as first input
  Multiply : Product  SaturateOnIntegerOverflow=on  RndMeth=Zero  InputSameDT=on  OutDataTypeStr=Inherit: Same as first input
  Ramp : simulink/Sources/Ramp  slope=FM  start=FT  X0=0
  Step : Step  Time=FT  Before=1  After=(1 + FM)  SampleTime=0
  StuckAt : Step  Time=FT  Before=1  After=0  SampleTime=0
  StuckAt2 : Step  Time=FT  After=FM  SampleTime=0
  Sum : Sum  SaturateOnIntegerOverflow=on  InputSameDT=on  OutDataTypeStr=Inherit: Same as first input
  Sum2 : Sum  SaturateOnIntegerOverflow=on  InputSameDT=on  OutDataTypeStr=Inherit: Same as first input
  Sum3 : Sum  SaturateOnIntegerOverflow=on  InputSameDT=on  OutDataTypeStr=Inherit: Same as first input
  E : Outport
  wires:
  Fault Inject/1(Out1) -> E/1
  Se/1 -> Fault Inject/1(Nominal), Multiply/1, Sum/1, Sum2/1, Mult2/1
  Fault Profile/1 -> Fault Inject/2(Fault Profile)
  Step/1 -> Multiply/2
  Ramp/1 -> Sum/2
  Multiply/1 -> Fault Inject/3(Step)
  Sum/1 -> Fault Inject/4(Ramp)
  Bias/1 -> Sum2/2
  Sum2/1 -> Fault Inject/5(Bias)
  StuckAt/1 -> Mult2/2
  Mult2/1 -> Sum3/1
  StuckAt2/1 -> Sum3/2
  Sum3/1 -> Fault Inject/6(stuckAt)

== system VirtualADAPT/VirtualADAPTv1/PowerStorage/RelayEY160/Sensor/R ==
  In : Inport
  Bond : Constant
  Computing R : Subsystem
  Fault Profile : Constant  Value=FP
  JuncDB : Constant  Value=2
  Junction Type : Constant  Value=0
  Ramp : simulink/Sources/Ramp  slope=FM  start=FT  X0=R
  Resistor Value : Constant  Value=R
  Step : Step  Time=FT  Before=R  After=(1 + FM)*R  SampleTime=0
  Out : Outport
  R : Outport  Port=2
  wires:
  Computing R/1(Out1) -> Out/1
  In/1 -> Computing R/1(In1)
  Resistor Value/1 -> Computing R/2(RNominal)
  Fault Profile/1 -> Computing R/3(Fault Profile)
  Step/1 -> Computing R/4(Step)
  Ramp/1 -> Computing R/5(Ramp)
  JuncDB/1 -> Computing R/6(JuncDB)
  Bond/1 -> Computing R/7(Bond)
  Junction Type/1 -> Computing R/8(Junction Type)
  Computing R/2(Out2) -> R/1

== system VirtualADAPT/VirtualADAPTv1/PowerStorage/RelayEY160/Sensor/TrueValueMod ==
  u(1) : Inport
  Function : Fcn  Expr=u(1)
  Mux : Mux  Inputs=1
  y(1) : Outport
  wires:
  u(1)/1 -> Mux/1
  Mux/1 -> Function/1
  Function/1 -> y(1)/1

== system VirtualADAPT/VirtualADAPTv1/PowerStorage/RelayEY160/Sensor/Value ==
  u(1) : Inport
  u(2) : Inport  Port=2
  Adder : Sum  Inputs=-  SaturateOnIntegerOverflow=on  InputSameDT=on  OutDataTypeStr=Inherit: Same as first input
  Gain : Gain  Gain=-1  SaturateOnIntegerOverflow=on  OutDataTypeStr=Inherit: Same as input  ParamDataTypeStr=Inherit: Same as input
  y(1) : Outport
  y(2) : Outport  Port=2
  O : Outport  Port=3
  wires:
  Adder/1 -> Gain/1
  u(2)/1 -> y(1)/1, O/1
  u(1)/1 -> Adder/1
  Gain/1 -> y(2)/1

== system VirtualADAPT/VirtualADAPTv1/PowerStorage/RelayEY260/ParallelConn/determineState ==
  In1 : Inport
  In2 : Inport  Port=2
  Fcn : Fcn  Expr=(!u(1)&&u(2)) || (u(1)&&(!u(3)))
  Memory : Memory
  Mux : Mux  Inputs=3
  Out1 : Outport
  wires:
  Memory/1 -> Mux/1
  In1/1 -> Mux/2
  In2/1 -> Mux/3
  Mux/1 -> Fcn/1
  Fcn/1 -> Memory/1, Out1/1

== system VirtualADAPT/VirtualADAPTv1/PowerStorage/RelayEY260/Relay/determineState ==
  In1 : Inport
  In2 : Inport  Port=2
  Fcn : Fcn  Expr=(!u(1)&&u(2)) || (u(1)&&(!u(3)))
  Memory : Memory
  Mux : Mux  Inputs=3
  Out1 : Outport
  wires:
  Memory/1 -> Mux/1
  In1/1 -> Mux/2
  In2/1 -> Mux/3
  Mux/1 -> Fcn/1
  Fcn/1 -> Memory/1, Out1/1

== system VirtualADAPT/VirtualADAPTv1/PowerStorage/RelayEY260/Sensor/MSe ==
  F : Inport
  Se : Inport  Port=2
  Bias : Step  Time=FT  After=FM  SampleTime=0
  Fault Inject : Subsystem
  Fault Profile : Constant  Value=FP
  Mult2 : Product  SaturateOnIntegerOverflow=on  RndMeth=Zero  InputSameDT=on  OutDataTypeStr=Inherit: Same as first input
  Multiply : Product  SaturateOnIntegerOverflow=on  RndMeth=Zero  InputSameDT=on  OutDataTypeStr=Inherit: Same as first input
  Ramp : simulink/Sources/Ramp  slope=FM  start=FT  X0=0
  Step : Step  Time=FT  Before=1  After=(1 + FM)  SampleTime=0
  StuckAt : Step  Time=FT  Before=1  After=0  SampleTime=0
  StuckAt2 : Step  Time=FT  After=FM  SampleTime=0
  Sum : Sum  SaturateOnIntegerOverflow=on  InputSameDT=on  OutDataTypeStr=Inherit: Same as first input
  Sum2 : Sum  SaturateOnIntegerOverflow=on  InputSameDT=on  OutDataTypeStr=Inherit: Same as first input
  Sum3 : Sum  SaturateOnIntegerOverflow=on  InputSameDT=on  OutDataTypeStr=Inherit: Same as first input
  E : Outport
  wires:
  Fault Inject/1(Out1) -> E/1
  Se/1 -> Fault Inject/1(Nominal), Multiply/1, Sum/1, Sum2/1, Mult2/1
  Fault Profile/1 -> Fault Inject/2(Fault Profile)
  Step/1 -> Multiply/2
  Ramp/1 -> Sum/2
  Multiply/1 -> Fault Inject/3(Step)
  Sum/1 -> Fault Inject/4(Ramp)
  Bias/1 -> Sum2/2
  Sum2/1 -> Fault Inject/5(Bias)
  StuckAt/1 -> Mult2/2
  Mult2/1 -> Sum3/1
  StuckAt2/1 -> Sum3/2
  Sum3/1 -> Fault Inject/6(stuckAt)

== system VirtualADAPT/VirtualADAPTv1/PowerStorage/RelayEY260/Sensor/R ==
  In : Inport
  Bond : Constant
  Computing R : Subsystem
  Fault Profile : Constant  Value=FP
  JuncDB : Constant  Value=2
  Junction Type : Constant  Value=0
  Ramp : simulink/Sources/Ramp  slope=FM  start=FT  X0=R
  Resistor Value : Constant  Value=R
  Step : Step  Time=FT  Before=R  After=(1 + FM)*R  SampleTime=0
  Out : Outport
  R : Outport  Port=2
  wires:
  Computing R/1(Out1) -> Out/1
  In/1 -> Computing R/1(In1)
  Resistor Value/1 -> Computing R/2(RNominal)
  Fault Profile/1 -> Computing R/3(Fault Profile)
  Step/1 -> Computing R/4(Step)
  Ramp/1 -> Computing R/5(Ramp)
  JuncDB/1 -> Computing R/6(JuncDB)
  Bond/1 -> Computing R/7(Bond)
  Junction Type/1 -> Computing R/8(Junction Type)
  Computing R/2(Out2) -> R/1

== system VirtualADAPT/VirtualADAPTv1/PowerStorage/RelayEY260/Sensor/TrueValueMod ==
  u(1) : Inport
  Function : Fcn  Expr=u(1)
  Mux : Mux  Inputs=1
  y(1) : Outport
  wires:
  u(1)/1 -> Mux/1
  Mux/1 -> Function/1
  Function/1 -> y(1)/1

== system VirtualADAPT/VirtualADAPTv1/PowerStorage/RelayEY260/Sensor/Value ==
  u(1) : Inport
  u(2) : Inport  Port=2
  Adder : Sum  Inputs=-  SaturateOnIntegerOverflow=on  InputSameDT=on  OutDataTypeStr=Inherit: Same as first input
  Gain : Gain  Gain=-1  SaturateOnIntegerOverflow=on  OutDataTypeStr=Inherit: Same as input  ParamDataTypeStr=Inherit: Same as input
  y(1) : Outport
  y(2) : Outport  Port=2
  O : Outport  Port=3
  wires:
  Adder/1 -> Gain/1
  u(2)/1 -> y(1)/1, O/1
  u(1)/1 -> Adder/1
  Gain/1 -> y(2)/1

== system VirtualADAPT/VirtualADAPTv1/PowerStorage/SensorE161/MSe/Fault Inject ==
  Nominal : Inport
  Fault Profile : Inport  Port=2
  Step : Inport  Port=3
  Ramp : Inport  Port=4
  Bias : Inport  Port=5
  stuckAt : Inport  Port=6
  Switch1 : MultiPortSwitch  Inputs=5  SaturateOnIntegerOverflow=on  InputSameDT=on
  Out1 : Outport
  wires:
  Nominal/1 -> Switch1/2
  Fault Profile/1 -> Switch1/1
  Step/1 -> Switch1/3
  Ramp/1 -> Switch1/4
  Bias/1 -> Switch1/5
  stuckAt/1 -> Switch1/6
  Switch1/1 -> Out1/1

== system VirtualADAPT/VirtualADAPTv1/PowerStorage/SensorE161/R/Computing R ==
  In1 : Inport
  RNominal : Inport  Port=2
  Fault Profile : Inport  Port=3
  Step : Inport  Port=4
  Ramp : Inport  Port=5
  JuncDB : Inport  Port=6
  Bond : Inport  Port=7
  Junction Type : Inport  Port=8
  CalcCausality : Fcn  Expr=(u(1) == u(2) && u(3) ==1) || (u(1) !=u(2) && u(3) == 0)
  CalcOutput : Fcn  Expr=(u(1)*u(2)^u(3))
  MinusOne : Constant  Value=-1
  Mux1 : Mux  Inputs=3
  Mux2 : Mux  Inputs=3
  One : Constant
  Switch1 : MultiPortSwitch  SaturateOnIntegerOverflow=on  InputSameDT=on
  Switch2 : MultiPortSwitch  DataPortOrder=Zero-based contiguous  Inputs=2  zeroidx=on  SaturateOnIntegerOverflow=on  InputSameDT=on
  Out1 : Outport
  Out2 : Outport  Port=2
  wires:
  In1/1 -> Mux2/1
  Fault Profile/1 -> Switch1/1
  RNominal/1 -> Switch1/2
  Step/1 -> Switch1/3
  Ramp/1 -> Switch1/4
  Switch1/1 -> Out2/1, Mux2/2
  JuncDB/1 -> Mux1/1
  Bond/1 -> Mux1/2
  Junction Type/1 -> Mux1/3
  Mux1/1 -> CalcCausality/1
  CalcCausality/1 -> Switch2/1
  One/1 -> Switch2/2
  MinusOne/1 -> Switch2/3
  Switch2/1 -> Mux2/3
  Mux2/1 -> CalcOutput/1
  CalcOutput/1 -> Out1/1

== system VirtualADAPT/VirtualADAPTv1/PowerStorage/SensorE261/MSe/Fault Inject ==
  Nominal : Inport
  Fault Profile : Inport  Port=2
  Step : Inport  Port=3
  Ramp : Inport  Port=4
  Bias : Inport  Port=5
  stuckAt : Inport  Port=6
  Switch1 : MultiPortSwitch  Inputs=5  SaturateOnIntegerOverflow=on  InputSameDT=on
  Out1 : Outport
  wires:
  Nominal/1 -> Switch1/2
  Fault Profile/1 -> Switch1/1
  Step/1 -> Switch1/3
  Ramp/1 -> Switch1/4
  Bias/1 -> Switch1/5
  stuckAt/1 -> Switch1/6
  Switch1/1 -> Out1/1

== system VirtualADAPT/VirtualADAPTv1/PowerStorage/SensorE261/R/Computing R ==
  In1 : Inport
  RNominal : Inport  Port=2
  Fault Profile : Inport  Port=3
  Step : Inport  Port=4
  Ramp : Inport  Port=5
  JuncDB : Inport  Port=6
  Bond : Inport  Port=7
  Junction Type : Inport  Port=8
  CalcCausality : Fcn  Expr=(u(1) == u(2) && u(3) ==1) || (u(1) !=u(2) && u(3) == 0)
  CalcOutput : Fcn  Expr=(u(1)*u(2)^u(3))
  MinusOne : Constant  Value=-1
  Mux1 : Mux  Inputs=3
  Mux2 : Mux  Inputs=3
  One : Constant
  Switch1 : MultiPortSwitch  SaturateOnIntegerOverflow=on  InputSameDT=on
  Switch2 : MultiPortSwitch  DataPortOrder=Zero-based contiguous  Inputs=2  zeroidx=on  SaturateOnIntegerOverflow=on  InputSameDT=on
  Out1 : Outport
  Out2 : Outport  Port=2
  wires:
  In1/1 -> Mux2/1
  Fault Profile/1 -> Switch1/1
  RNominal/1 -> Switch1/2
  Step/1 -> Switch1/3
  Ramp/1 -> Switch1/4
  Switch1/1 -> Out2/1, Mux2/2
  JuncDB/1 -> Mux1/1
  Bond/1 -> Mux1/2
  Junction Type/1 -> Mux1/3
  Mux1/1 -> CalcCausality/1
  CalcCausality/1 -> Switch2/1
  One/1 -> Switch2/2
  MinusOne/1 -> Switch2/3
  Switch2/1 -> Mux2/3
  Mux2/1 -> CalcOutput/1
  CalcOutput/1 -> Out1/1

== system VirtualADAPT/VirtualADAPTv1/PowerStorage/SensorIT161/MSe/Fault Inject ==
  Nominal : Inport
  Fault Profile : Inport  Port=2
  Step : Inport  Port=3
  Ramp : Inport  Port=4
  Bias : Inport  Port=5
  stuckAt : Inport  Port=6
  Switch1 : MultiPortSwitch  Inputs=5  SaturateOnIntegerOverflow=on  InputSameDT=on
  Out1 : Outport
  wires:
  Nominal/1 -> Switch1/2
  Fault Profile/1 -> Switch1/1
  Step/1 -> Switch1/3
  Ramp/1 -> Switch1/4
  Bias/1 -> Switch1/5
  stuckAt/1 -> Switch1/6
  Switch1/1 -> Out1/1

== system VirtualADAPT/VirtualADAPTv1/PowerStorage/SensorIT161/R/Computing R ==
  In1 : Inport
  RNominal : Inport  Port=2
  Fault Profile : Inport  Port=3
  Step : Inport  Port=4
  Ramp : Inport  Port=5
  JuncDB : Inport  Port=6
  Bond : Inport  Port=7
  Junction Type : Inport  Port=8
  CalcCausality : Fcn  Expr=(u(1) == u(2) && u(3) ==1) || (u(1) !=u(2) && u(3) == 0)
  CalcOutput : Fcn  Expr=(u(1)*u(2)^u(3))
  MinusOne : Constant  Value=-1
  Mux1 : Mux  Inputs=3
  Mux2 : Mux  Inputs=3
  One : Constant
  Switch1 : MultiPortSwitch  SaturateOnIntegerOverflow=on  InputSameDT=on
  Switch2 : MultiPortSwitch  DataPortOrder=Zero-based contiguous  Inputs=2  zeroidx=on  SaturateOnIntegerOverflow=on  InputSameDT=on
  Out1 : Outport
  Out2 : Outport  Port=2
  wires:
  In1/1 -> Mux2/1
  Fault Profile/1 -> Switch1/1
  RNominal/1 -> Switch1/2
  Step/1 -> Switch1/3
  Ramp/1 -> Switch1/4
  Switch1/1 -> Out2/1, Mux2/2
  JuncDB/1 -> Mux1/1
  Bond/1 -> Mux1/2
  Junction Type/1 -> Mux1/3
  Mux1/1 -> CalcCausality/1
  CalcCausality/1 -> Switch2/1
  One/1 -> Switch2/2
  MinusOne/1 -> Switch2/3
  Switch2/1 -> Mux2/3
  Mux2/1 -> CalcOutput/1
  CalcOutput/1 -> Out1/1

== system VirtualADAPT/VirtualADAPTv1/PowerStorage/SensorIT261/MSe/Fault Inject ==
  Nominal : Inport
  Fault Profile : Inport  Port=2
  Step : Inport  Port=3
  Ramp : Inport  Port=4
  Bias : Inport  Port=5
  stuckAt : Inport  Port=6
  Switch1 : MultiPortSwitch  Inputs=5  SaturateOnIntegerOverflow=on  InputSameDT=on
  Out1 : Outport
  wires:
  Nominal/1 -> Switch1/2
  Fault Profile/1 -> Switch1/1
  Step/1 -> Switch1/3
  Ramp/1 -> Switch1/4
  Bias/1 -> Switch1/5
  stuckAt/1 -> Switch1/6
  Switch1/1 -> Out1/1

== system VirtualADAPT/VirtualADAPTv1/PowerStorage/SensorIT261/R/Computing R ==
  In1 : Inport
  RNominal : Inport  Port=2
  Fault Profile : Inport  Port=3
  Step : Inport  Port=4
  Ramp : Inport  Port=5
  JuncDB : Inport  Port=6
  Bond : Inport  Port=7
  Junction Type : Inport  Port=8
  CalcCausality : Fcn  Expr=(u(1) == u(2) && u(3) ==1) || (u(1) !=u(2) && u(3) == 0)
  CalcOutput : Fcn  Expr=(u(1)*u(2)^u(3))
  MinusOne : Constant  Value=-1
  Mux1 : Mux  Inputs=3
  Mux2 : Mux  Inputs=3
  One : Constant
  Switch1 : MultiPortSwitch  SaturateOnIntegerOverflow=on  InputSameDT=on
  Switch2 : MultiPortSwitch  DataPortOrder=Zero-based contiguous  Inputs=2  zeroidx=on  SaturateOnIntegerOverflow=on  InputSameDT=on
  Out1 : Outport
  Out2 : Outport  Port=2
  wires:
  In1/1 -> Mux2/1
  Fault Profile/1 -> Switch1/1
  RNominal/1 -> Switch1/2
  Step/1 -> Switch1/3
  Ramp/1 -> Switch1/4
  Switch1/1 -> Out2/1, Mux2/2
  JuncDB/1 -> Mux1/1
  Bond/1 -> Mux1/2
  Junction Type/1 -> Mux1/3
  Mux1/1 -> CalcCausality/1
  CalcCausality/1 -> Switch2/1
  One/1 -> Switch2/2
  MinusOne/1 -> Switch2/3
  Switch2/1 -> Mux2/3
  Mux2/1 -> CalcOutput/1
  CalcOutput/1 -> Out1/1

== system VirtualADAPT/VirtualADAPTv1/PowerDistribution/ACLoadBank1/L1A/LGT6/ComputeCurrent ==
  u(1) : Inport
  u(2) : Inport  Port=2
  u(3) : Inport  Port=3
  Function : Fcn  Expr=u(1)+u(2)+u(3)
  Mux : Mux  Inputs=3
  y(1) : Outport
  wires:
  u(1)/1 -> Mux/1
  u(2)/1 -> Mux/2
  u(3)/1 -> Mux/3
  Mux/1 -> Function/1
  Function/1 -> y(1)/1

== system VirtualADAPT/VirtualADAPTv1/PowerDistribution/ACLoadBank1/L1A/LGT6/ComputePower ==
  u(1) : Inport
  u(2) : Inport  Port=2
  u(3) : Inport  Port=3
  Function : Fcn  Expr=(u(1)+u(2)+u(3))
  Mux : Mux  Inputs=3
  y(1) : Outport
  wires:
  u(1)/1 -> Mux/1
  u(2)/1 -> Mux/2
  u(3)/1 -> Mux/3
  Mux/1 -> Function/1
  Function/1 -> y(1)/1
